simulink model "s_c856m1kfloat_v2"  (R2016b)
settings: Solver=VariableStepAuto  StartTime=0.0  StopTime=1024*10  RelTol=1e-3  EnableMultiTasking=on
values from neighbouring files (symbols this model uses):
  fft_stages = 13    [s_ct_workspace.mat]
  output_bits = 8    [s_ct_workspace.mat]
  xengbits = 4    [s_ct_workspace.mat]
libraries: xbsIndex_r4 x22437, casper_library_misc x39, casper_library_delays x13, xps_library x3, xbsReplacements_r4 x3
(part 48 of 172)

== system s_c856m1kfloat_v2/pfb/pfb_fir_generic/bus_expand ==
  bus_in : Inport
  reinterpret{1..16} (16 alike) : xbsIndex_r4/Reinterpret  force_arith_type=on  arith_type="Signed  (2's comp)"  force_bin_pt=on  bin_pt=17  block_type=reinterpret
  slice1 : xbsIndex_r4/Slice  nbits=18  boolean_output=off  mode=Upper Bit Location + Width  bit1=-270  base1=MSB of Input  bit0=0  base0=LSB of Input  dbl_ovrd=off  block_type=slice
  slice10 : xbsIndex_r4/Slice  nbits=18  boolean_output=off  mode=Upper Bit Location + Width  bit1=-108  base1=MSB of Input  bit0=0  base0=LSB of Input  dbl_ovrd=off  block_type=slice
  slice11 : xbsIndex_r4/Slice  nbits=18  boolean_output=off  mode=Upper Bit Location + Width  bit1=-90  base1=MSB of Input  bit0=0  base0=LSB of Input  dbl_ovrd=off  block_type=slice
  slice12 : xbsIndex_r4/Slice  nbits=18  boolean_output=off  mode=Upper Bit Location + Width  bit1=-72  base1=MSB of Input  bit0=0  base0=LSB of Input  dbl_ovrd=off  block_type=slice
  slice13 : xbsIndex_r4/Slice  nbits=18  boolean_output=off  mode=Upper Bit Location + Width  bit1=-54  base1=MSB of Input  bit0=0  base0=LSB of Input  dbl_ovrd=off  block_type=slice
  slice14 : xbsIndex_r4/Slice  nbits=18  boolean_output=off  mode=Upper Bit Location + Width  bit1=-36  base1=MSB of Input  bit0=0  base0=LSB of Input  dbl_ovrd=off  block_type=slice
  slice15 : xbsIndex_r4/Slice  nbits=18  boolean_output=off  mode=Upper Bit Location + Width  bit1=-18  base1=MSB of Input  bit0=0  base0=LSB of Input  dbl_ovrd=off  block_type=slice
  slice16 : xbsIndex_r4/Slice  nbits=18  boolean_output=off  mode=Upper Bit Location + Width  bit1=0  base1=MSB of Input  bit0=0  base0=LSB of Input  dbl_ovrd=off  block_type=slice
  slice2 : xbsIndex_r4/Slice  nbits=18  boolean_output=off  mode=Upper Bit Location + Width  bit1=-252  base1=MSB of Input  bit0=0  base0=LSB of Input  dbl_ovrd=off  block_type=slice
  slice3 : xbsIndex_r4/Slice  nbits=18  boolean_output=off  mode=Upper Bit Location + Width  bit1=-234  base1=MSB of Input  bit0=0  base0=LSB of Input  dbl_ovrd=off  block_type=slice
  slice4 : xbsIndex_r4/Slice  nbits=18  boolean_output=off  mode=Upper Bit Location + Width  bit1=-216  base1=MSB of Input  bit0=0  base0=LSB of Input  dbl_ovrd=off  block_type=slice
  slice5 : xbsIndex_r4/Slice  nbits=18  boolean_output=off  mode=Upper Bit Location + Width  bit1=-198  base1=MSB of Input  bit0=0  base0=LSB of Input  dbl_ovrd=off  block_type=slice
  slice6 : xbsIndex_r4/Slice  nbits=18  boolean_output=off  mode=Upper Bit Location + Width  bit1=-180  base1=MSB of Input  bit0=0  base0=LSB of Input  dbl_ovrd=off  block_type=slice
  slice7 : xbsIndex_r4/Slice  nbits=18  boolean_output=off  mode=Upper Bit Location + Width  bit1=-162  base1=MSB of Input  bit0=0  base0=LSB of Input  dbl_ovrd=off  block_type=slice
  slice8 : xbsIndex_r4/Slice  nbits=18  boolean_output=off  mode=Upper Bit Location + Width  bit1=-144  base1=MSB of Input  bit0=0  base0=LSB of Input  dbl_ovrd=off  block_type=slice
  slice9 : xbsIndex_r4/Slice  nbits=18  boolean_output=off  mode=Upper Bit Location + Width  bit1=-126  base1=MSB of Input  bit0=0  base0=LSB of Input  dbl_ovrd=off  block_type=slice
  msb_out16 : Outport
  out15 : Outport  Port=2
  out14 : Outport  Port=3
  out13 : Outport  Port=4
  out12 : Outport  Port=5
  out11 : Outport  Port=6
  out10 : Outport  Port=7
  out9 : Outport  Port=8
  out8 : Outport  Port=9
  out7 : Outport  Port=10
  out6 : Outport  Port=11
  out5 : Outport  Port=12
  out4 : Outport  Port=13
  out3 : Outport  Port=14
  out2 : Outport  Port=15
  lsb_out1 : Outport  Port=16
  wires:
  bus_in/1 -> slice16/1, slice15/1, slice14/1, slice13/1, slice12/1, slice11/1, slice10/1, slice9/1, slice8/1, slice7/1, slice6/1, slice5/1, slice4/1, slice3/1, slice2/1, slice1/1
  slice16/1 -> reinterpret16/1
  reinterpret16/1 -> msb_out16/1
  slice15/1 -> reinterpret15/1
  reinterpret15/1 -> out15/1
  slice14/1 -> reinterpret14/1
  reinterpret14/1 -> out14/1
  slice13/1 -> reinterpret13/1
  reinterpret13/1 -> out13/1
  slice12/1 -> reinterpret12/1
  reinterpret12/1 -> out12/1
  slice11/1 -> reinterpret11/1
  reinterpret11/1 -> out11/1
  slice10/1 -> reinterpret10/1
  reinterpret10/1 -> out10/1
  slice9/1 -> reinterpret9/1
  reinterpret9/1 -> out9/1
  slice8/1 -> reinterpret8/1
  reinterpret8/1 -> out8/1
  slice7/1 -> reinterpret7/1
  reinterpret7/1 -> out7/1
  slice6/1 -> reinterpret6/1
  reinterpret6/1 -> out6/1
  slice5/1 -> reinterpret5/1
  reinterpret5/1 -> out5/1
  slice4/1 -> reinterpret4/1
  reinterpret4/1 -> out4/1
  slice3/1 -> reinterpret3/1
  reinterpret3/1 -> out3/1
  slice2/1 -> reinterpret2/1
  reinterpret2/1 -> out2/1
  slice1/1 -> reinterpret1/1
  reinterpret1/1 -> lsb_out1/1

== system s_c856m1kfloat_v2/pfb/pfb_fir_generic/bus_scale ==
  din : Inport
  bussify : Subsystem  AncestorBlock=casper_library_flow_control/bus_create  mask(bus_create){inputNum=16}
      mask prompts: inputNum "Number of inputs:"
      mask init:
      | bus_create_init(gcb,...
      |     'inputNum', inputNum);
  debus : Subsystem  AncestorBlock=casper_library_flow_control/bus_expand  mask(bus_expand){mode=divisions of arbitrary size, outputNum=1, outputWidth=[31 31 31 31 31 31 31 31 31 31 31 31 31 31 31 31], outputBinaryPt=[26 26 26 26 26 26 26 26 26 26 26 26 26 26 26 26], outputArithmeticType=[1 1 1 1 1 1 1 1 1 1 1 1 1 1 1 1], show_format=on, outputToWorkspace=off, variablePrefix=, outputToModelAsWell=on}
      mask prompts: outputNum "Number of outputs:"; outputWidth "Output width [msb ... lsb]:"; outputBinaryPt "Output binary point position [msb ... lsb]:"; outputArithmeticType "Output arithmetic type (ufix=0, fix=1, bool=2) [msb ... lsb]:"; show_format "Print format string?"; outputToWorkspace "Output to workspace?"; variablePrefix "Variable name prefix:"; outputToModelAsWell "Output to model as well?"
      mask help:
      | Expands a 'bus' made using a bus_create or Xilinx
      | concat block.
      | The input will be divided into the specified number
      | of outputs with the specified arithmetic types.
      | Divisions are made from most significant bit.
      mask init: same as s_c856m1kfloat_v2/bus_expand2
      settings: AttributesFormatString=16 outputs: f31.26,f31.26,f31.26,f31.26,f31.26,f31.26,f31.26,f31.26,f31.26,f31.26,f31.26,f31.26,f31.26,f31.26,f31.26,f31.26,
      mask TypeOptions mode: divisions of equal size | divisions of arbitrary size
      mask Callback mode: bus_expand_callback();
      mask Callback outputWidth: bus_expand_callback();
      mask Callback outputToWorkspace: bus_expand_callback();
  scale{1..16} (16 alike) : xbsIndex_r4/Scale  scale_factor=-1  dbl_ovrd=off  block_type=scale
  dout : Outport
  wires:
  din/1 -> debus/1(bus_in)
  debus/1(msb_out16) -> scale1/1
  debus/2(out15) -> scale2/1
  debus/3(out14) -> scale3/1
  debus/4(out13) -> scale4/1
  debus/5(out12) -> scale5/1
  debus/6(out11) -> scale6/1
  debus/7(out10) -> scale7/1
  debus/8(out9) -> scale8/1
  debus/9(out8) -> scale9/1
  debus/10(out7) -> scale10/1
  debus/11(out6) -> scale11/1
  debus/12(out5) -> scale12/1
  debus/13(out4) -> scale13/1
  debus/14(out3) -> scale14/1
  debus/15(out2) -> scale15/1
  debus/16(lsb_out1) -> scale16/1
  scale1/1 -> bussify/1(in1)
  scale2/1 -> bussify/2(in2)
  scale3/1 -> bussify/3(in3)
  scale4/1 -> bussify/4(in4)
  scale5/1 -> bussify/5(in5)
  scale6/1 -> bussify/6(in6)
  scale7/1 -> bussify/7(in7)
  scale8/1 -> bussify/8(in8)
  scale9/1 -> bussify/9(in9)
  scale10/1 -> bussify/10(in10)
  scale11/1 -> bussify/11(in11)
  scale12/1 -> bussify/12(in12)
  scale13/1 -> bussify/13(in13)
  scale14/1 -> bussify/14(in14)
  scale15/1 -> bussify/15(in15)
  scale16/1 -> bussify/16(in16)
  bussify/1(bus_out) -> dout/1

== system s_c856m1kfloat_v2/pfb/pfb_fir_generic/coeff_munge ==
  din : Inport
  join : Subsystem  AncestorBlock=casper_library_flow_control/bus_create  mask(bus_create){inputNum=128}
      mask prompts: inputNum "Number of inputs:"
      mask init:
      | bus_create_init(gcb,...
      |     'inputNum', inputNum);
  reinterpret : xbsIndex_r4/Reinterpret  force_arith_type=on  arith_type=Unsigned  force_bin_pt=on  bin_pt=0  block_type=reinterpret
  reinterpret_out : xbsIndex_r4/Reinterpret  force_arith_type=on  arith_type=Unsigned  force_bin_pt=on  bin_pt=0  block_type=reinterpret
  split : Subsystem  AncestorBlock=casper_library_flow_control/bus_expand  mask(bus_expand){mode=divisions of arbitrary size, outputNum=1, outputWidth=[18 18 18 18 18 18 18 18 18 18 18 18 18 18 18 18 18 18 18 18 18 18 18 18 18 18 18 18 18 18 18 18 18 18 18 18 18 18 18 18 18 18 18 18 18 18 18 18 18 18 18 18 18 18 18 18 18 18 18 18 18 18 18 18], outputBinaryPt=[0 0 0 0 0 0 0 0 0 0 0 0 0 0 0 0 0 0 0 0 0 0 0 0 0 0 0 0 0 0 0 0 0 0 0 0 0 0 0 0 0 0 0 0 0 0 0 0 0 0 0 0 0 0 0 0 0 0 0 0 0 0 0 0], outputArithmeticType=[0 0 0 0 0 0 0 0 0 0 0 0 0 0 0 0 0 0 0 0 0 0 0 0 0 0 0 0 0 0 0 0 0 0 0 0 0 0 0 0 0 0 0 0 0 0 0 0 0 0 0 0 0 0 0 0 0 0 0 0 0 0 0 0], show_format=off, outputToWorkspace=off, variablePrefix=out, outputToModelAsWell=on}
      mask prompts: outputNum "Number of outputs:"; outputWidth "Output width [msb ... lsb]:"; outputBinaryPt "Output binary point position [msb ... lsb]:"; outputArithmeticType "Output arithmetic type (ufix=0, fix=1, bool=2) [msb ... lsb]:"; show_format "Print format string?"; outputToWorkspace "Output to workspace?"; variablePrefix "Variable name prefix:"; outputToModelAsWell "Output to model as well?"
      mask help:
      | Expands a 'bus' made using a bus_create or Xilinx
      | concat block.
      | The input will be divided into the specified number
      | of outputs with the specified arithmetic types.
      | Divisions are made from most significant bit.
      mask init: same as s_c856m1kfloat_v2/bus_expand2
      settings: AttributesFormatString=64 outputs
      mask TypeOptions mode: divisions of equal size | divisions of arbitrary size
      mask Callback mode: bus_expand_callback();
      mask Callback outputWidth: bus_expand_callback();
      mask Callback outputToWorkspace: bus_expand_callback();
  dout : Outport
  wires:
  reinterpret_out/1 -> dout/1
  din/1 -> reinterpret/1
  reinterpret/1 -> split/1(bus_in)
  join/1(bus_out) -> reinterpret_out/1
  split/57(out8) -> join/1(in1), join/2(in2)
  split/58(out7) -> join/3(in3), join/4(in4)
  split/59(out6) -> join/5(in5), join/6(in6)
  split/60(out5) -> join/7(in7), join/8(in8)
  split/61(out4) -> join/9(in9), join/10(in10)
  split/62(out3) -> join/11(in11), join/12(in12)
  split/63(out2) -> join/13(in13), join/14(in14)
  split/64(lsb_out1) -> join/15(in15), join/16(in16)
  split/49(out16) -> join/17(in17), join/18(in18)
  split/50(out15) -> join/19(in19), join/20(in20)
  split/51(out14) -> join/21(in21), join/22(in22)
  split/52(out13) -> join/23(in23), join/24(in24)
  split/53(out12) -> join/25(in25), join/26(in26)
  split/54(out11) -> join/27(in27), join/28(in28)
  split/55(out10) -> join/29(in29), join/30(in30)
  split/56(out9) -> join/31(in31), join/32(in32)
  split/41(out24) -> join/33(in33), join/34(in34)
  split/42(out23) -> join/35(in35), join/36(in36)
  split/43(out22) -> join/37(in37), join/38(in38)
  split/44(out21) -> join/39(in39), join/40(in40)
  split/45(out20) -> join/41(in41), join/42(in42)
  split/46(out19) -> join/43(in43), join/44(in44)
  split/47(out18) -> join/45(in45), join/46(in46)
  split/48(out17) -> join/47(in47), join/48(in48)
  split/33(out32) -> join/49(in49), join/50(in50)
  split/34(out31) -> join/51(in51), join/52(in52)
  split/35(out30) -> join/53(in53), join/54(in54)
  split/36(out29) -> join/55(in55), join/56(in56)
  split/37(out28) -> join/57(in57), join/58(in58)
  split/38(out27) -> join/59(in59), join/60(in60)
  split/39(out26) -> join/61(in61), join/62(in62)
  split/40(out25) -> join/63(in63), join/64(in64)
  split/25(out40) -> join/65(in65), join/66(in66)
  split/26(out39) -> join/67(in67), join/68(in68)
  split/27(out38) -> join/69(in69), join/70(in70)
  split/28(out37) -> join/71(in71), join/72(in72)
  split/29(out36) -> join/73(in73), join/74(in74)
  split/30(out35) -> join/75(in75), join/76(in76)
  split/31(out34) -> join/77(in77), join/78(in78)
  split/32(out33) -> join/79(in79), join/80(in80)
  split/17(out48) -> join/81(in81), join/82(in82)
  split/18(out47) -> join/83(in83), join/84(in84)
  split/19(out46) -> join/85(in85), join/86(in86)
  split/20(out45) -> join/87(in87), join/88(in88)
  split/21(out44) -> join/89(in89), join/90(in90)
  split/22(out43) -> join/91(in91), join/92(in92)
  split/23(out42) -> join/93(in93), join/94(in94)
  split/24(out41) -> join/95(in95), join/96(in96)
  split/9(out56) -> join/97(in97), join/98(in98)
  split/10(out55) -> join/99(in99), join/100(in100)
  split/11(out54) -> join/101(in101), join/102(in102)
  split/12(out53) -> join/103(in103), join/104(in104)
  split/13(out52) -> join/105(in105), join/106(in106)
  split/14(out51) -> join/107(in107), join/108(in108)
  split/15(out50) -> join/109(in109), join/110(in110)
  split/16(out49) -> join/111(in111), join/112(in112)
  split/1(msb_out64) -> join/113(in113), join/114(in114)
  split/2(out63) -> join/115(in115), join/116(in116)
  split/3(out62) -> join/117(in117), join/118(in118)
  split/4(out61) -> join/119(in119), join/120(in120)
  split/5(out60) -> join/121(in121), join/122(in122)
  split/6(out59) -> join/123(in123), join/124(in124)
  split/7(out58) -> join/125(in125), join/126(in126)
  split/8(out57) -> join/127(in127), join/128(in128)

== system s_c856m1kfloat_v2/pfb/pfb_fir_generic/pfb_fir_coeff_gen ==
  sync : Inport
  din : Inport  Port=2
  en : Inport  Port=3
  a_expand : Subsystem  AncestorBlock=casper_library_flow_control/bus_expand  mask(bus_expand){mode=divisions of equal size, outputNum=32, outputWidth=18, outputBinaryPt=0, outputArithmeticType=0, show_format=off, outputToWorkspace=off, variablePrefix=out, outputToModelAsWell=on}
      mask prompts: outputNum "Number of outputs:"; outputWidth "Output width:"; outputBinaryPt "Output binary point position:"; outputArithmeticType "Output arithmetic type (ufix=0, fix=1, bool=2):"; show_format "Print format string?"; outputToWorkspace "Output to workspace?"; variablePrefix "Variable name prefix:"; outputToModelAsWell "Output to model as well?"
      mask help:
      | Expands a 'bus' made using a bus_create or Xilinx
      | concat block.
      | The input will be divided into the specified number
      | of outputs with the specified arithmetic types.
      | Divisions are made from most significant bit.
      mask init: same as s_c856m1kfloat_v2/bus_expand2
      settings: AttributesFormatString=32 outputs
      mask TypeOptions mode: divisions of equal size | divisions of arbitrary size
      mask Callback mode: bus_expand_callback();
      mask Callback outputWidth: bus_expand_callback();
      mask Callback outputToWorkspace: bus_expand_callback();
  b_expand : Subsystem  AncestorBlock=casper_library_flow_control/bus_expand  mask(bus_expand){mode=divisions of equal size, outputNum=32, outputWidth=18, outputBinaryPt=0, outputArithmeticType=0, show_format=off, outputToWorkspace=off, variablePrefix=out, outputToModelAsWell=on}
      mask prompts: outputNum "Number of outputs:"; outputWidth "Output width:"; outputBinaryPt "Output binary point position:"; outputArithmeticType "Output arithmetic type (ufix=0, fix=1, bool=2):"; show_format "Print format string?"; outputToWorkspace "Output to workspace?"; variablePrefix "Variable name prefix:"; outputToModelAsWell "Output to model as well?"
      mask help:
      | Expands a 'bus' made using a bus_create or Xilinx
      | concat block.
      | The input will be divided into the specified number
      | of outputs with the specified arithmetic types.
      | Divisions are made from most significant bit.
      mask init: same as s_c856m1kfloat_v2/bus_expand2
      settings: AttributesFormatString=32 outputs
      mask TypeOptions mode: divisions of equal size | divisions of arbitrary size
      mask Callback mode: bus_expand_callback();
      mask Callback outputWidth: bus_expand_callback();
      mask Callback outputToWorkspace: bus_expand_callback();
  concat : xbsIndex_r4/Concat  num_inputs=64  dbl_ovrd=off  block_type=concat
  const0 : xbsIndex_r4/Constant  const=1  gui_display_data_type=Fixed-point  arith_type=Signed (2's comp)  n_bits=18  bin_pt=17  preci_type=Single  exp_width=8  frac_width=24  explicit_period=on  period=1  equ=P=C  opselect=C  inp2=PCIN>>17  opr=+  inp1=P  carry=CIN  dbl_ovrd=off  block_type=constant
  const1 : xbsIndex_r4/Constant  const=2.7116e-17  gui_display_data_type=Fixed-point  arith_type=Signed (2's comp)  n_bits=18  bin_pt=17  preci_type=Single  exp_width=8  frac_width=24  explicit_period=on  period=1  equ=P=C  opselect=C  inp2=PCIN>>17  opr=+  inp1=P  carry=CIN  dbl_ovrd=off  block_type=constant
  const2 : xbsIndex_r4/Constant  const=-8.4723e-18  gui_display_data_type=Fixed-point  arith_type=Signed (2's comp)  n_bits=18  bin_pt=17  preci_type=Single  exp_width=8  frac_width=24  explicit_period=on  period=1  equ=P=C  opselect=C  inp2=PCIN>>17  opr=+  inp1=P  carry=CIN  dbl_ovrd=off  block_type=constant
  const3 : xbsIndex_r4/Constant  const=8.4616e-19  gui_display_data_type=Fixed-point  arith_type=Signed (2's comp)  n_bits=18  bin_pt=17  preci_type=Single  exp_width=8  frac_width=24  explicit_period=on  period=1  equ=P=C  opselect=C  inp2=PCIN>>17  opr=+  inp1=P  carry=CIN  dbl_ovrd=off  block_type=constant
  counter : xbsIndex_r4/Counter  cnt_type=Free Running  cnt_to=Inf  operation=Up  start_count=0  cnt_by_val=1  arith_type=Unsigned  n_bits=8  bin_pt=0  load_pin=off  rst=on  en=on  explicit_period=on  period=1  use_behavioral_HDL=off  implementation=Fabric  dbl_ovrd=off  block_type=counter
  d{0..31,33..39,41..47,49..55,57..63} (60 alike) : xbsIndex_r4/Delay  rst=off  infoeditControl=Selection of Reset will increase slice count due to use of real FFs and instead of SRLs  en=off  latency=1  reg_retiming=on  dbl_ovrd=off  block_type=delay
      settings: infoedit=Hardware notes: A delay line is a chain, each link of which is an SRL16 or SRL32 followed by a flip-flop.
  d{32,40,48,56} (4 alike) : xbsIndex_r4/Delay  rst=off  infoeditControl=Selection of Reset will increase slice count due to use of real FFs and instead of SRLs  en=on  latency=1  reg_retiming=on  dbl_ovrd=off  block_type=delay
      settings: infoedit=Hardware notes: A delay line is a chain, each link of which is an SRL16 or SRL32 followed by a flip-flop.
  den0 : xbsIndex_r4/Delay  rst=off  infoeditControl=Selection of Reset will increase slice count due to use of real FFs and instead of SRLs  en=off  latency=8  reg_retiming=on  dbl_ovrd=off  block_type=delay
      settings: infoedit=Hardware notes: A delay line is a chain, each link of which is an SRL16 or SRL32 followed by a flip-flop.
  den1 : xbsIndex_r4/Delay  rst=off  infoeditControl=Selection of Reset will increase slice count due to use of real FFs and instead of SRLs  en=off  latency=1  reg_retiming=on  dbl_ovrd=off  block_type=delay
      settings: infoedit=Hardware notes: A delay line is a chain, each link of which is an SRL16 or SRL32 followed by a flip-flop.
  dfirst : xbsIndex_r4/Delay  rst=off  infoeditControl=Selection of Reset will increase slice count due to use of real FFs and instead of SRLs  en=off  latency=4  reg_retiming=on  dbl_ovrd=off  block_type=delay
      settings: infoedit=Hardware notes: A delay line is a chain, each link of which is an SRL16 or SRL32 followed by a flip-flop.
  din_delay : xbsIndex_r4/Delay  rst=off  infoeditControl=Selection of Reset will increase slice count due to use of real FFs and instead of SRLs  en=off  latency=9  reg_retiming=on  dbl_ovrd=off  block_type=delay
      settings: infoedit=Hardware notes: A delay line is a chain, each link of which is an SRL16 or SRL32 followed by a flip-flop.
  first : xbsIndex_r4/Relational  mode=a=b  op_type=Bool  en=off  latency=4  dbl_ovrd=off  block_type=relational
  inverter : xbsIndex_r4/Inverter  en=off  latency=0  dbl_ovrd=off  block_type=inv
  munge : Subsystem  AncestorBlock=casper_library_flow_control/munge  mask(munge){divisions=32, div_size=[18 18 18 18 18 18 18 18 18 18 18 18 18 18 18 18 18 18 18 18 18 18 18 18 18 18 18 18 18 18 18 18], order=[24 31 30 29 28 27 26 25 16 23 22 21 20 19 18 17 8 15 14 13 12 11 10 9 0 7 6 5 4 3 2 1], arith_type_out=Unsigned, bin_pt_out=0}
      mask prompts: divisions "Number of divisions"; div_size "Division size (bits)"; order "Division packing order"; arith_type_out "Output arithmetic type"; bin_pt_out "Output binary point"
      mask help: Splits an input bus into the specified number of divisions and then recombines them in the order specified (most significant division = 0,least = number divisions-1)
      mask init:
      | munge_init(gcb, ...
      |     'divisions', divisions, ...
      |     'div_size', div_size, ...
      |     'order', order, ...
      |     'arith_type_out', arith_type_out, ...
      |     'bin_pt_out', bin_pt_out);
      settings: AttributesFormatString=|
      | split:[18 18 18 18 18 18 18 18 18 18 18 18 18 18 18 18 18 18 18 18 18 18 18 18 18 18 18 18 18 18 18 18]
      | join:[24 31 30 29 28 27 26 25 16 23 22 21 20 19 18 17 8 15 14 13 12 11 10 9 0 7 6 5 4 3 2 1]
      | Unsigned [576,0]
      mask TypeOptions arith_type_out: Unsigned | Signed  (2's comp) | Floating-point
  mux{0..3} (4 alike) : xbsIndex_r4/Mux  inputs=2  en=off  latency=1  precision=Full  arith_type=Unsigned  n_bits=16  bin_pt=14  quantization=Truncate  overflow=Wrap  dbl_ovrd=off  block_type=mux
  reinterpret{0..3} (4 alike) : xbsIndex_r4/Reinterpret  force_arith_type=on  arith_type=Unsigned  force_bin_pt=on  bin_pt=0  block_type=reinterpret
  rom : Subsystem  AncestorBlock=casper_library_bus/bus_dual_port_ram  mask(bus_dual_port_ram){n_bits=[18 18 18 18 18 18 18 18 18 18 18 18 18 18 18 18 18 18 18 18 18 18 18 18 18 18 18 18 18 18 18 18], bin_pts=[17 17 17 17 17 17 17 17 17 17 17 17 17 17 17 17 17 17 17 17 17 17 17 17 17 17 17 17 17 17 17 17], init_vector=[pfb_coeff_gen_calc(11, 8, 'blackmanharris', 3, 0, 1, 1, false)', pfb_coeff_gen_calc(11, 8, 'blackmanharris', 3, 1, 1, 1, false)', pfb_coeff_gen_calc(11, 8, 'blackmanharris', 3, 2, 1, 1, false)', pfb_coeff_gen_calc(11, 8, 'blackmanharris', 3, 3, 1, 1, false)', pfb_coeff_gen_calc(11, 8, 'blackmanharris', 3, 4, 1, 1, false)', pfb_coeff_gen_calc(11, 8, 'blackmanharris', 3, 5, 1, 1, false)', pfb_coeff_gen_calc(11, 8, 'blackmanharris', 3, 6, 1, 1, false)', pfb_coeff_gen_calc(11, 8, 'blackmanharris', 3, 7, 1, 1, false)', pfb_coeff_gen_calc(11, 8, 'blackmanharris', 3, 0, 1, 2, false)', pfb_coeff_gen_calc(11, 8, 'blackmanharris', 3, 1, 1, 2, false)', pfb_coeff_gen_calc(11, 8, 'blackmanharris', 3, 2, 1, 2, false)', pfb_coeff_gen_calc(11, 8, 'blackmanharris', 3, 3, 1, 2, false)', pfb_coeff_gen_calc(11, 8, 'blackmanharris', 3, 4, 1, 2, false)', pfb_coeff_gen_calc(11, 8, 'blackmanharris', 3, 5, 1, 2, false)', pfb_coeff_gen_calc(11, 8, 'blackmanharris', 3, 6, 1, 2, false)', pfb_coeff_gen_calc(11, 8, 'blackmanharris', 3, 7, 1, 2, false)', pfb_coeff_gen_calc(11, 8, 'blackmanharris', 3, 0, 1, 3, false)', pfb_coeff_gen_calc(11, 8, 'blackmanharris', 3, 1, 1, 3, false)', pfb_coeff_gen_calc(11, 8, 'blackmanharris', 3, 2, 1, 3, false)', pfb_coeff_gen_calc(11, 8, 'blackmanharris', 3, 3, 1, 3, false)', pfb_coeff_gen_calc(11, 8, 'blackmanharris', 3, 4, 1, 3, false)', pfb_coeff_gen_calc(11, 8, 'blackmanharris', 3, 5, 1, 3, false)', pfb_coeff_gen_calc(11, 8, 'blackmanharris', 3, 6, 1, 3, false)', pfb_coeff_gen_calc(11, 8, 'blackmanharris', 3, 7, 1, 3, false)', pfb_coeff_gen_calc(11, 8, 'blackmanharris', 3, 0, 1, 4, false)', pfb_coeff_gen_calc(11, 8, 'blackmanharris', 3, 1, 1, 4, false)', pfb_coeff_gen_calc(11, 8, 'blackmanharris', 3, 2, 1, 4, false)', pfb_coeff_gen_calc(11, 8, 'blackmanharris', 3, 3, 1, 4, false)', pfb_coeff_gen_calc(11, 8, 'blackmanharris', 3, 4, 1, 4, false)', pfb_coeff_gen_calc(11, 8, 'blackmanharris', 3, 5, 1, 4, false)', pfb_coeff_gen_calc(11, 8, 'blackmanharris', 3, 6, 1, 4, false)', pfb_coeff_gen_calc(11, 8, 'blackmanharris', 3, 7, 1, 4, false)'], max_fanout=1, mem_type=Block RAM, bram_optimization=Speed, async_a=off, async_b=off, b_to_a_ratio_bits=0, misc=off, bram_latency=4, fan_latency=4, addra_register=on, addra_implementation=core, dina_register=off, dina_implementation=behavioral, wea_register=off, wea_implementation=behavioral, ena_register=off, ena_implementation=core, addrb_register=on, addrb_implementation=core, dinb_register=off, dinb_implementation=behavioral, web_register=off, web_implementation=behavioral, enb_register=off, enb_implementation=core}
      mask prompts: n_bits "data word bit widths"; bin_pts "data word binary points"; init_vector "initial value vector"; max_fanout "limit fanout to ?"; mem_type "memory type"; bram_optimization "memory optimization"; async_a "a asynchronous"; async_b "b asynchronous"; b_to_a_ratio_bits "b_to_a ratio bits"; misc "misc support"; bram_latency "bram latency"; fan_latency "input register latency"; addra_register "addra input register"; addra_implementation "addra register implementation"; dina_register "dina input register"; dina_implementation "dina register implementation"; wea_register "wea input register"; wea_implementation "wea register implementation"; ena_register "ena input register"; ena_implementation "ena register implementation"; addrb_register "addrb input register"; addrb_implementation "addrb register implementation"; dinb_register "dinb input register"; dinb_implementation "dinb register implementation"; web_register "web input register"; web_implementation "web register implementation"; enb_register "enb input register"; enb_implementation "enb register implementation"
      mask help: RAM for a bus allowing fanout control
      mask init:
      | bus_dual_port_ram_init(gcb, ...
      |     'n_bits', n_bits, ...
      |     'bin_pts', bin_pts, ...
      |     'init_vector', init_vector, ...
      |     'max_fanout', max_fanout, ...
      |     'mem_type', mem_type, ...
      |     'bram_optimization', bram_optimization, ...
      |     'async_a', async_a, ...
      |     'async_b', async_b, ...
      |     'b_to_a_ratio_bits', b_to_a_ratio_bits, ...
      |     'misc', misc, ...
      |     'bram_latency', bram_latency, ...
      |     'fan_latency', fan_latency, ...
      |     'addra_register', addra_register, ...
      |     'addra_implementation', addra_implementation, ...
      |     'dina_register', dina_register, ...
      |     'dina_implementation', dina_implementation, ...
      |     'wea_register', wea_register, ...
      |     'wea_implementation', wea_implementation, ...
      |     'ena_register', ena_register, ...
      |     'ena_implementation', ena_implementation, ...
      |     'addrb_register', addrb_register, ...
      |     'addrb_implementation', addrb_implementation, ...
      |     'dinb_register', dinb_register, ...
      |     'dinb_implementation', dinb_implementation, ...
      |     'web_register', web_register, ...
      |     'web_implementation', web_implementation, ...
      |     'enb_register', enb_register, ...
      |     'enb_implementation', enb_implementation);
      mask TypeOptions mem_type: Distributed memory | Block RAM
      mask TypeOptions bram_optimization: Area | Speed
      mask TypeOptions addra_implementation: core | behavioral
      mask TypeOptions dina_implementation: core | behavioral
      mask TypeOptions wea_implementation: core | behavioral
      mask TypeOptions ena_implementation: core | behavioral
      mask TypeOptions addrb_implementation: core | behavioral
      mask TypeOptions dinb_implementation: core | behavioral
      mask TypeOptions web_implementation: core | behavioral
      mask TypeOptions enb_implementation: core | behavioral
  rom_din : xbsIndex_r4/Constant  const=0  gui_display_data_type=Fixed-point  arith_type=Unsigned  n_bits=576  bin_pt=0  preci_type=Single  exp_width=8  frac_width=24  explicit_period=on  period=1  equ=P=C  opselect=C  inp2=PCIN>>17  opr=+  inp1=P  carry=CIN  dbl_ovrd=off  block_type=constant
  rom_we : xbsIndex_r4/Constant  const=0  gui_display_data_type=Fixed-point  arith_type=Boolean  n_bits=16  bin_pt=14  preci_type=Single  exp_width=8  frac_width=24  explicit_period=on  period=1  equ=P=C  opselect=C  inp2=PCIN>>17  opr=+  inp1=P  carry=CIN  dbl_ovrd=off  block_type=constant
  sync_delay : xbsIndex_r4/Delay  rst=off  infoeditControl=Selection of Reset will increase slice count due to use of real FFs and instead of SRLs  en=off  latency=9  reg_retiming=on  dbl_ovrd=off  block_type=delay
      settings: infoedit=Hardware notes: A delay line is a chain, each link of which is an SRL16 or SRL32 followed by a flip-flop.
  zero : xbsIndex_r4/Constant  const=0  gui_display_data_type=Fixed-point  arith_type=Unsigned  n_bits=8  bin_pt=0  preci_type=Single  exp_width=8  frac_width=24  explicit_period=on  period=1  equ=P=C  opselect=C  inp2=PCIN>>17  opr=+  inp1=P  carry=CIN  dbl_ovrd=off  block_type=constant
  sync_out : Outport
  dout : Outport  Port=2
  coeffs : Outport  Port=3
  dvalid : Outport  Port=4
  wires:
  sync/1 -> sync_delay/1, counter/1
  sync_delay/1 -> sync_out/1
  din/1 -> din_delay/1
  din_delay/1 -> dout/1
  counter/1 -> inverter/1, rom/1(addra), first/2
  inverter/1 -> rom/4(addrb)
  rom_din/1 -> rom/2(dina), rom/5(dinb)
  rom_we/1 -> rom/3(wea), rom/6(web)
  zero/1 -> first/1
  first/1 -> dfirst/1
  rom/2(B) -> munge/1(din)
  rom/1(A) -> a_expand/1(bus_in)
  munge/1(dout) -> b_expand/1(bus_in)
  concat/1 -> coeffs/1
  a_expand/1(msb_out32) -> d0/1
  d0/1 -> concat/1
  a_expand/2(out31) -> d1/1
  d1/1 -> concat/2
  a_expand/3(out30) -> d2/1
  d2/1 -> concat/3
  a_expand/4(out29) -> d3/1
  d3/1 -> concat/4
  a_expand/5(out28) -> d4/1
  d4/1 -> concat/5
  a_expand/6(out27) -> d5/1
  d5/1 -> concat/6
  a_expand/7(out26) -> d6/1
  d6/1 -> concat/7
  a_expand/8(out25) -> d7/1
  d7/1 -> concat/8
  a_expand/9(out24) -> d8/1
  d8/1 -> concat/9
  a_expand/10(out23) -> d9/1
  d9/1 -> concat/10
  a_expand/11(out22) -> d10/1
  d10/1 -> concat/11
  a_expand/12(out21) -> d11/1
  d11/1 -> concat/12
  a_expand/13(out20) -> d12/1
  d12/1 -> concat/13
  a_expand/14(out19) -> d13/1
  d13/1 -> concat/14
  a_expand/15(out18) -> d14/1
  d14/1 -> concat/15
  a_expand/16(out17) -> d15/1
  d15/1 -> concat/16
  a_expand/17(out16) -> d16/1
  d16/1 -> concat/17
  a_expand/18(out15) -> d17/1
  d17/1 -> concat/18
  a_expand/19(out14) -> d18/1
  d18/1 -> concat/19
  a_expand/20(out13) -> d19/1
  d19/1 -> concat/20
  a_expand/21(out12) -> d20/1
  d20/1 -> concat/21
  a_expand/22(out11) -> d21/1
  d21/1 -> concat/22
  a_expand/23(out10) -> d22/1
  d22/1 -> concat/23
  a_expand/24(out9) -> d23/1
  d23/1 -> concat/24
  a_expand/25(out8) -> d24/1
  d24/1 -> concat/25
  a_expand/26(out7) -> d25/1
  d25/1 -> concat/26
  a_expand/27(out6) -> d26/1
  d26/1 -> concat/27
  a_expand/28(out5) -> d27/1
  d27/1 -> concat/28
  a_expand/29(out4) -> d28/1
  d28/1 -> concat/29
  a_expand/30(out3) -> d29/1
  d29/1 -> concat/30
  a_expand/31(out2) -> d30/1
  d30/1 -> concat/31
  a_expand/32(lsb_out1) -> d31/1
  d31/1 -> concat/32
  b_expand/1(msb_out32) -> d32/1
  const0/1 -> reinterpret0/1
  reinterpret0/1 -> mux0/3
  dfirst/1 -> mux0/1, mux1/1, mux2/1, mux3/1
  d32/1 -> mux0/2
  mux0/1 -> concat/33
  b_expand/2(out31) -> d33/1
  d33/1 -> concat/34
  b_expand/3(out30) -> d34/1
  d34/1 -> concat/35
  b_expand/4(out29) -> d35/1
  d35/1 -> concat/36
  b_expand/5(out28) -> d36/1
  d36/1 -> concat/37
  b_expand/6(out27) -> d37/1
  d37/1 -> concat/38
  b_expand/7(out26) -> d38/1
  d38/1 -> concat/39
  b_expand/8(out25) -> d39/1
  d39/1 -> concat/40
  b_expand/9(out24) -> d40/1
  const1/1 -> reinterpret1/1
  reinterpret1/1 -> mux1/3
  d40/1 -> mux1/2
  mux1/1 -> concat/41
  b_expand/10(out23) -> d41/1
  d41/1 -> concat/42
  b_expand/11(out22) -> d42/1
  d42/1 -> concat/43
  b_expand/12(out21) -> d43/1
  d43/1 -> concat/44
  b_expand/13(out20) -> d44/1
  d44/1 -> concat/45
  b_expand/14(out19) -> d45/1
  d45/1 -> concat/46
  b_expand/15(out18) -> d46/1
  d46/1 -> concat/47
  b_expand/16(out17) -> d47/1
  d47/1 -> concat/48
  b_expand/17(out16) -> d48/1
  const2/1 -> reinterpret2/1
  reinterpret2/1 -> mux2/3
  d48/1 -> mux2/2
  mux2/1 -> concat/49
  b_expand/18(out15) -> d49/1
  d49/1 -> concat/50
  b_expand/19(out14) -> d50/1
  d50/1 -> concat/51
  b_expand/20(out13) -> d51/1
  d51/1 -> concat/52
  b_expand/21(out12) -> d52/1
  d52/1 -> concat/53
  b_expand/22(out11) -> d53/1
  d53/1 -> concat/54
  b_expand/23(out10) -> d54/1
  d54/1 -> concat/55
  b_expand/24(out9) -> d55/1
  d55/1 -> concat/56
  b_expand/25(out8) -> d56/1
  const3/1 -> reinterpret3/1
  reinterpret3/1 -> mux3/3
  d56/1 -> mux3/2
  mux3/1 -> concat/57
  b_expand/26(out7) -> d57/1
  d57/1 -> concat/58
  b_expand/27(out6) -> d58/1
  d58/1 -> concat/59
  b_expand/28(out5) -> d59/1
  d59/1 -> concat/60
  b_expand/29(out4) -> d60/1
  d60/1 -> concat/61
  b_expand/30(out3) -> d61/1
  d61/1 -> concat/62
  b_expand/31(out2) -> d62/1
  d62/1 -> concat/63
  b_expand/32(lsb_out1) -> d63/1
  d63/1 -> concat/64
  en/1 -> counter/2, den0/1
  den0/1 -> d32/2, d40/2, d48/2, d56/2, den1/1
  den1/1 -> dvalid/1
